simulink model "doa_diego"  (R2013b)
settings: Solver=ode45  StartTime=0.0  StopTime=10.0  RelTol=1e-3
undefined symbols (not defined in the file or in its folder): calan_lib, comp_addsub, pfb_coeff_gen_calc
libraries: xbsIndex_r4 x26847, casper_library_misc x201, casper_library_delays x104, dspsigops x16, xbsReplacements_r4 x4, casper_library_pfbs x4, simulink x2, xps_library x1
(part 2 of 280)

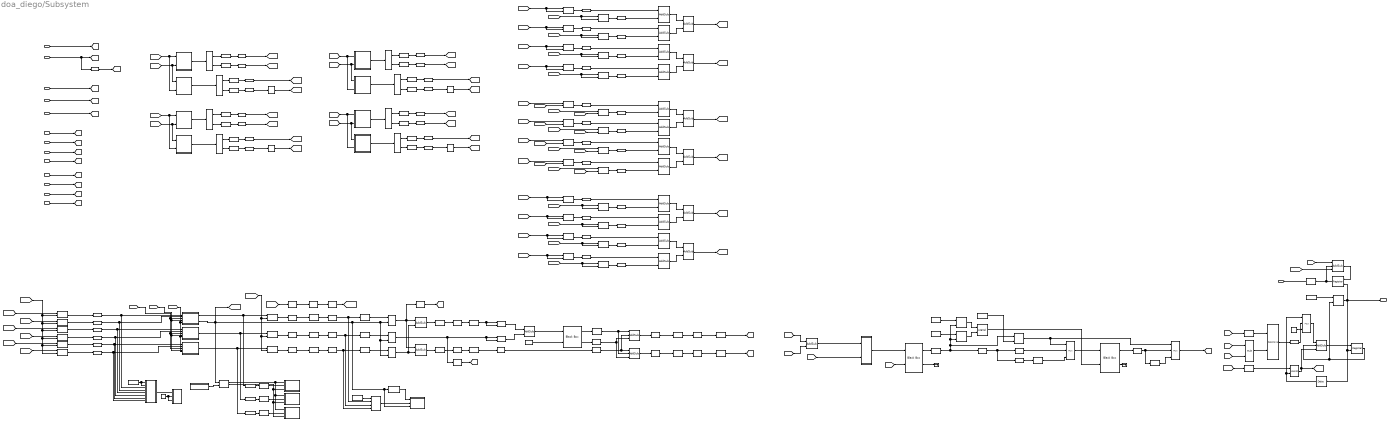
== system doa_diego/Subsystem ==
  gain1 : Inport
  gain2 : Inport  Port=2
  nacc : Inport  Port=3
  snr : Inport  Port=4
  en : Inport  Port=5
  a1_ch0 : Inport  Port=6
  a1_ch1 : Inport  Port=7
  a1_ch2 : Inport  Port=8
  a1_ch3 : Inport  Port=9
  a2_ch0 : Inport  Port=10
  a2_ch1 : Inport  Port=11
  a2_ch2 : Inport  Port=12
  a2_ch3 : Inport  Port=13
  AddSub : xbsIndex_r4/AddSub  mode=Addition  use_carryin=off  use_carryout=off  en=on  latency=1  precision=User Defined  arith_type="Signed  (2's comp)"  n_bits=26  bin_pt=8  quantization="Round  (unbiased: +/- Inf)"  overflow=Saturate  dbl_ovrd=off  use_behavioral_HDL=off  hw_selection=Fabric  pipelined=off  block_type=addsub
  AddSub1 : xbsIndex_r4/AddSub  mode=Addition  use_carryin=off  use_carryout=off  en=on  latency=1  precision=User Defined  arith_type=Unsigned  n_bits=10  bin_pt=0  quantization="Round  (unbiased: +/- Inf)"  overflow=Saturate  dbl_ovrd=off  use_behavioral_HDL=off  hw_selection=Fabric  pipelined=off  block_type=addsub
  AddSub{2..5,8..11,14..15,18..19} (12 alike) : xbsIndex_r4/AddSub  mode=Addition  use_carryin=off  use_carryout=off  en=off  latency=0  precision=Full  arith_type="Signed  (2's comp)"  n_bits=9  bin_pt=7  quantization="Round  (unbiased: +/- Inf)"  overflow=Saturate  dbl_ovrd=off  use_behavioral_HDL=off  hw_selection=Fabric  pipelined=off  block_type=addsub
  AddSub{6..7,12..13,16..17} (6 alike) : xbsIndex_r4/AddSub  mode=Addition  use_carryin=off  use_carryout=off  en=off  latency=0  precision=Full  arith_type="Signed  (2's comp)"  n_bits=10  bin_pt=7  quantization="Round  (unbiased: +/- Inf)"  overflow=Saturate  dbl_ovrd=off  use_behavioral_HDL=off  hw_selection=Fabric  pipelined=off  block_type=addsub
  Black Box : xbsIndex_r4/Black Box  init_code=arcsin_config  sim_method=ISE Simulator  verbose=off  block_type=blackbox2
  Constant : xbsIndex_r4/Constant  const=0  gui_display_data_type=Fixed-point  arith_type=Unsigned  n_bits=8  bin_pt=0  preci_type=Single  exp_width=8  frac_width=24  explicit_period=on  period=1  equ=P=C  opselect=C  inp2=PCIN>>17  opr=+  inp1=P  carry=CIN  dbl_ovrd=off  block_type=constant
  Constant1 : xbsIndex_r4/Constant  const=1  gui_display_data_type=Boolean  arith_type=Boolean  n_bits=16  bin_pt=14  preci_type=Single  exp_width=8  frac_width=24  explicit_period=on  period=1  equ=P=C  opselect=C  inp2=PCIN>>17  opr=+  inp1=P  carry=CIN  dbl_ovrd=off  block_type=constant
  Constant16 : xbsIndex_r4/Constant  const=0  gui_display_data_type=Fixed-point  arith_type=Signed (2's comp)  n_bits=16  bin_pt=12  preci_type=Single  exp_width=8  frac_width=24  explicit_period=on  period=1  equ=P=C  opselect=C  inp2=PCIN>>17  opr=+  inp1=P  carry=CIN  dbl_ovrd=off  block_type=constant
  Constant17 : xbsIndex_r4/Constant  const=16  gui_display_data_type=Fixed-point  arith_type=Unsigned  n_bits=10  bin_pt=0  preci_type=Single  exp_width=8  frac_width=24  explicit_period=on  period=1  equ=P=C  opselect=C  inp2=PCIN>>17  opr=+  inp1=P  carry=CIN  dbl_ovrd=off  block_type=constant
  Constant18 : xbsIndex_r4/Constant  const=0  gui_display_data_type=Fixed-point  arith_type=Unsigned  n_bits=1  bin_pt=0  preci_type=Single  exp_width=8  frac_width=24  explicit_period=on  period=1  equ=P=C  opselect=C  inp2=PCIN>>17  opr=+  inp1=P  carry=CIN  dbl_ovrd=off  block_type=constant
  Constant2 : xbsIndex_r4/Constant  const=0  gui_display_data_type=Fixed-point  arith_type=Signed (2's comp)  n_bits=8  bin_pt=7  preci_type=Single  exp_width=8  frac_width=24  explicit_period=on  period=1  equ=P=C  opselect=C  inp2=PCIN>>17  opr=+  inp1=P  carry=CIN  dbl_ovrd=off  block_type=constant
  Constant6 : xbsIndex_r4/Constant  const=1  gui_display_data_type=Boolean  arith_type=Boolean  n_bits=16  bin_pt=14  preci_type=Single  exp_width=8  frac_width=24  explicit_period=off  period=1  equ=P=C  opselect=C  inp2=PCIN>>17  opr=+  inp1=P  carry=CIN  dbl_ovrd=off  block_type=constant
  Constant7 : xbsIndex_r4/Constant  const=1  gui_display_data_type=Fixed-point  arith_type=Signed (2's comp)  n_bits=16  bin_pt=12  preci_type=Single  exp_width=8  frac_width=24  explicit_period=on  period=1  equ=P=C  opselect=C  inp2=PCIN>>17  opr=+  inp1=P  carry=CIN  dbl_ovrd=off  block_type=constant
  Constant9 : xbsIndex_r4/Constant  const=-1  gui_display_data_type=Fixed-point  arith_type=Signed (2's comp)  n_bits=16  bin_pt=12  preci_type=Single  exp_width=8  frac_width=24  explicit_period=on  period=1  equ=P=C  opselect=C  inp2=PCIN>>17  opr=+  inp1=P  carry=CIN  dbl_ovrd=off  block_type=constant
  Convert{,1..7,15,29..34,41,46..49,54,59..64} (27 alike) : xbsIndex_r4/Convert  gui_display_data_type=Fixed-point  arith_type="Signed  (2's comp)"  n_bits=8  bin_pt=7  float_type=Single  exp_bits=8  fraction_bits=24  quantization="Round  (unbiased: +/- Inf)"  overflow=Saturate  en=off  latency=0  dbl_ovrd=off  pipeline=off  block_type=convert
  Convert10 : xbsIndex_r4/Convert  gui_display_data_type=Fixed-point  arith_type="Signed  (2's comp)"  n_bits=32  bin_pt=7  float_type=Single  exp_bits=8  fraction_bits=24  quantization=Truncate  overflow=Wrap  en=off  latency=0  dbl_ovrd=off  pipeline=off  block_type=convert
      settings: block_version=11.4
  Convert{11..14,20..25,66..75,82..83,93..94} (24 alike) : xbsIndex_r4/Convert  gui_display_data_type=Fixed-point  arith_type="Signed  (2's comp)"  n_bits=15  bin_pt=14  float_type=Single  exp_bits=8  fraction_bits=24  quantization="Round  (unbiased: +/- Inf)"  overflow=Saturate  en=off  latency=0  dbl_ovrd=off  pipeline=off  block_type=convert
  Convert16 : xbsIndex_r4/Convert  gui_display_data_type=Fixed-point  arith_type="Signed  (2's comp)"  n_bits=32  bin_pt=7  float_type=Single  exp_bits=8  fraction_bits=24  quantization=Truncate  overflow=Wrap  en=off  latency=0  dbl_ovrd=off  pipeline=off  block_type=convert
      settings: block_version=11.4
  Convert26 : xbsIndex_r4/Convert  gui_display_data_type=Fixed-point  arith_type="Signed  (2's comp)"  n_bits=15  bin_pt=6  float_type=Single  exp_bits=8  fraction_bits=24  quantization="Round  (unbiased: +/- Inf)"  overflow=Saturate  en=off  latency=0  dbl_ovrd=off  pipeline=off  block_type=convert
  Convert27 : xbsIndex_r4/Convert  gui_display_data_type=Fixed-point  arith_type="Signed  (2's comp)"  n_bits=9  bin_pt=8  float_type=Single  exp_bits=8  fraction_bits=24  quantization="Round  (unbiased: +/- Inf)"  overflow=Saturate  en=off  latency=0  dbl_ovrd=off  pipeline=off  block_type=convert
  Convert28 : xbsIndex_r4/Convert  gui_display_data_type=Fixed-point  arith_type="Signed  (2's comp)"  n_bits=13  bin_pt=12  float_type=Single  exp_bits=8  fraction_bits=24  quantization="Round  (unbiased: +/- Inf)"  overflow=Saturate  en=off  latency=0  dbl_ovrd=off  pipeline=off  block_type=convert
  Convert35 : xbsIndex_r4/Convert  gui_display_data_type=Fixed-point  arith_type="Signed  (2's comp)"  n_bits=28  bin_pt=7  float_type=Single  exp_bits=8  fraction_bits=24  quantization="Round  (unbiased: +/- Inf)"  overflow=Saturate  en=off  latency=0  dbl_ovrd=off  pipeline=off  block_type=convert
  Convert36 : xbsIndex_r4/Convert  gui_display_data_type=Fixed-point  arith_type="Signed  (2's comp)"  n_bits=28  bin_pt=7  float_type=Single  exp_bits=8  fraction_bits=24  quantization="Round  (unbiased: +/- Inf)"  overflow=Saturate  en=off  latency=0  dbl_ovrd=off  pipeline=off  block_type=convert
  Convert37 : xbsIndex_r4/Convert  gui_display_data_type=Fixed-point  arith_type="Signed  (2's comp)"  n_bits=28  bin_pt=7  float_type=Single  exp_bits=8  fraction_bits=24  quantization="Round  (unbiased: +/- Inf)"  overflow=Saturate  en=off  latency=0  dbl_ovrd=off  pipeline=off  block_type=convert
  Convert38 : xbsIndex_r4/Convert  gui_display_data_type=Fixed-point  arith_type="Signed  (2's comp)"  n_bits=15  bin_pt=6  float_type=Single  exp_bits=8  fraction_bits=24  quantization="Round  (unbiased: +/- Inf)"  overflow=Saturate  en=off  latency=0  dbl_ovrd=off  pipeline=off  block_type=convert
  Convert39 : xbsIndex_r4/Convert  gui_display_data_type=Fixed-point  arith_type="Signed  (2's comp)"  n_bits=9  bin_pt=8  float_type=Single  exp_bits=8  fraction_bits=24  quantization="Round  (unbiased: +/- Inf)"  overflow=Saturate  en=off  latency=0  dbl_ovrd=off  pipeline=off  block_type=convert
  Convert40 : xbsIndex_r4/Convert  gui_display_data_type=Fixed-point  arith_type=Unsigned  n_bits=1  bin_pt=0  float_type=Single  exp_bits=8  fraction_bits=24  quantization="Round  (unbiased: +/- Inf)"  overflow=Saturate  en=off  latency=0  dbl_ovrd=off  pipeline=off  block_type=convert
  Convert8 : xbsIndex_r4/Convert  gui_display_data_type=Boolean  arith_type=Boolean  n_bits=8  bin_pt=0  float_type=Single  exp_bits=8  fraction_bits=24  quantization="Round  (unbiased: +/- Inf)"  overflow=Saturate  en=off  latency=0  dbl_ovrd=off  pipeline=off  block_type=convert
  Convert9 : xbsIndex_r4/Convert  gui_display_data_type=Fixed-point  arith_type="Signed  (2's comp)"  n_bits=32  bin_pt=7  float_type=Single  exp_bits=8  fraction_bits=24  quantization=Truncate  overflow=Wrap  en=off  latency=0  dbl_ovrd=off  pipeline=off  block_type=convert
      settings: block_version=11.4
  Counter : xbsIndex_r4/Counter  cnt_type=Free Running  cnt_to=Inf  operation=Up  start_count=0  cnt_by_val=1  arith_type=Unsigned  n_bits=10  bin_pt=0  load_pin=off  rst=off  en=on  explicit_period=on  period=1  dbl_ovrd=off  use_behavioral_HDL=off  implementation=Fabric  block_type=counter
  Delay : xbsIndex_r4/Delay  rst=off  infoeditControl=Selection of Reset will increase slice count due to use of real FFs and instead of SRLs  en=off  latency=3  dbl_ovrd=off  reg_retiming=off  block_type=delay
  Delay1 : xbsIndex_r4/Delay  rst=off  infoeditControl=Selection of Reset will increase slice count due to use of real FFs and instead of SRLs  en=off  latency=1  dbl_ovrd=off  reg_retiming=off  block_type=delay
  Delay11 : xbsIndex_r4/Delay  rst=off  infoeditControl=Selection of Reset will increase slice count due to use of real FFs and instead of SRLs  en=off  latency=3  dbl_ovrd=off  reg_retiming=off  block_type=delay
  Delay{4,14,16,20..21} (5 alike) : xbsIndex_r4/Delay  rst=off  infoeditControl=Selection of Reset will increase slice count due to use of real FFs and instead of SRLs  en=off  latency=4  dbl_ovrd=off  reg_retiming=off  block_type=delay
  Delay2 : xbsIndex_r4/Delay  rst=off  infoeditControl=Selection of Reset will increase slice count due to use of real FFs and instead of SRLs  en=off  latency=3  dbl_ovrd=off  reg_retiming=off  block_type=delay
  Delay22 : xbsIndex_r4/Delay  rst=off  infoeditControl=Selection of Reset will increase slice count due to use of real FFs and instead of SRLs  en=off  latency=1  dbl_ovrd=off  reg_retiming=off  block_type=delay
  Logical : xbsIndex_r4/Logical  logical_function=OR  inputs=2  en=off  latency=0  precision=Full  arith_type=Unsigned  n_bits=16  bin_pt=0  align_bp=on  dbl_ovrd=off  block_type=logical
  Logical1 : xbsIndex_r4/Logical  logical_function=AND  inputs=2  en=off  latency=0  precision=Full  arith_type=Unsigned  n_bits=16  bin_pt=0  align_bp=on  dbl_ovrd=off  block_type=logical
  Mult{,0..11,14..34} (34 alike) : xbsIndex_r4/Mult  precision=Full  arith_type="Signed  (2's comp)"  n_bits=16  bin_pt=14  quantization=Truncate  overflow=Wrap  en=off  latency=3  dbl_ovrd=off  use_behavioral_HDL=off  opt=Speed  use_embedded=on  optimum_pipeline=off  pipeline=on  use_rpm=on  placement_style=Triangular  block_type=mult
  Mult12 : xbsIndex_r4/Mult  precision=User Defined  arith_type=Unsigned  n_bits=16  bin_pt=0  quantization="Round  (unbiased: +/- Inf)"  overflow=Saturate  en=off  latency=3  dbl_ovrd=off  use_behavioral_HDL=off  opt=Speed  use_embedded=on  optimum_pipeline=off  pipeline=on  use_rpm=on  placement_style=Triangular  block_type=mult
  Mult13 : xbsIndex_r4/Mult  precision=User Defined  arith_type=Unsigned  n_bits=16  bin_pt=0  quantization="Round  (unbiased: +/- Inf)"  overflow=Saturate  en=off  latency=3  dbl_ovrd=off  use_behavioral_HDL=off  opt=Speed  use_embedded=on  optimum_pipeline=off  pipeline=on  use_rpm=on  placement_style=Triangular  block_type=mult
  Mux : xbsIndex_r4/Mux  inputs=2  en=off  latency=0  precision=Full  arith_type=Unsigned  n_bits=16  bin_pt=14  quantization=Truncate  overflow=Wrap  dbl_ovrd=off  block_type=mux
  Mux1 : xbsIndex_r4/Mux  inputs=2  en=off  latency=0  precision=Full  arith_type=Unsigned  n_bits=16  bin_pt=14  quantization=Truncate  overflow=Wrap  dbl_ovrd=off  block_type=mux
  Mux2 : xbsIndex_r4/Mux  inputs=2  en=off  latency=0  precision=Full  arith_type=Unsigned  n_bits=16  bin_pt=14  quantization=Truncate  overflow=Wrap  dbl_ovrd=off  block_type=mux
  Mx11 : xbsIndex_r4/Register  init=0  rst=off  en=on  dbl_ovrd=off  block_type=register
  Mx12 : xbsIndex_r4/Register  init=0  rst=off  en=on  dbl_ovrd=off  block_type=register
  Mx22 : xbsIndex_r4/Register  init=0  rst=off  en=on  dbl_ovrd=off  block_type=register
  Negate{,4,6,8} (4 alike) : xbsIndex_r4/Negate  precision=User Defined  arith_type="Signed  (2's comp)"  n_bits=8  bin_pt=7  quantization="Round  (unbiased: +/- Inf)"  overflow=Saturate  en=off  latency=0  dbl_ovrd=off  block_type=negate
  Negate2 : xbsIndex_r4/Negate  precision=User Defined  arith_type="Signed  (2's comp)"  n_bits=13  bin_pt=12  quantization="Round  (unbiased: +/- Inf)"  overflow=Saturate  en=off  latency=0  dbl_ovrd=off  block_type=negate
  Negate3 : xbsIndex_r4/Negate  precision=User Defined  arith_type="Signed  (2's comp)"  n_bits=16  bin_pt=8  quantization="Round  (unbiased: +/- Inf)"  overflow=Saturate  en=off  latency=0  dbl_ovrd=off  block_type=negate
  Register : xbsIndex_r4/Register  init=0  rst=on  en=off  dbl_ovrd=off  block_type=register
  Register1 : xbsIndex_r4/Register  init=0  rst=on  en=off  dbl_ovrd=off  block_type=register
  Reinterpret : xbsIndex_r4/Reinterpret  force_arith_type=off  arith_type=Unsigned  force_bin_pt=on  bin_pt=6  block_type=reinterpret
  Reinterpret1 : xbsIndex_r4/Reinterpret  force_arith_type=off  arith_type=Unsigned  force_bin_pt=off  bin_pt=0  block_type=reinterpret
  Reinterpret10 : xbsIndex_r4/Reinterpret  force_arith_type=on  arith_type="Signed  (2's comp)"  force_bin_pt=on  bin_pt=0  block_type=reinterpret
  Reinterpret{7..9,11..14,22..23,37..41,43..45} (17 alike) : xbsIndex_r4/Reinterpret  force_arith_type=on  arith_type="Signed  (2's comp)"  force_bin_pt=on  bin_pt=8  block_type=reinterpret
  Reinterpret15 : xbsIndex_r4/Reinterpret  force_arith_type=off  arith_type=Unsigned  force_bin_pt=on  bin_pt=14  block_type=reinterpret
  Reinterpret{6,16,21,42} (4 alike) : xbsIndex_r4/Reinterpret  force_arith_type=off  arith_type=Unsigned  force_bin_pt=on  bin_pt=0  block_type=reinterpret
  Reinterpret17 : xbsIndex_r4/Reinterpret  force_arith_type=on  arith_type="Signed  (2's comp)"  force_bin_pt=on  bin_pt=12  block_type=reinterpret
  Reinterpret18 : xbsIndex_r4/Reinterpret  force_arith_type=on  arith_type=Unsigned  force_bin_pt=on  bin_pt=0  block_type=reinterpret
  Reinterpret19 : xbsIndex_r4/Reinterpret  force_arith_type=on  arith_type=Unsigned  force_bin_pt=on  bin_pt=0  block_type=reinterpret
  Reinterpret2 : xbsIndex_r4/Reinterpret  force_arith_type=off  arith_type=Unsigned  force_bin_pt=off  bin_pt=0  block_type=reinterpret
  Reinterpret20 : xbsIndex_r4/Reinterpret  force_arith_type=off  arith_type=Unsigned  force_bin_pt=on  bin_pt=14  block_type=reinterpret
  Reinterpret3 : xbsIndex_r4/Reinterpret  force_arith_type=off  arith_type=Unsigned  force_bin_pt=off  bin_pt=0  block_type=reinterpret
  Reinterpret30 : xbsIndex_r4/Reinterpret  force_arith_type=off  arith_type=Unsigned  force_bin_pt=on  bin_pt=8  block_type=reinterpret
  Reinterpret5 : xbsIndex_r4/Reinterpret  force_arith_type=off  arith_type=Unsigned  force_bin_pt=on  bin_pt=8  block_type=reinterpret
  Reinterpret53 : xbsIndex_r4/Reinterpret  force_arith_type=on  arith_type="Signed  (2's comp)"  force_bin_pt=on  bin_pt=27  block_type=reinterpret
  Reinterpret64 : xbsIndex_r4/Reinterpret  force_arith_type=on  arith_type="Signed  (2's comp)"  force_bin_pt=on  bin_pt=0  block_type=reinterpret
  Reinterpret75 : xbsIndex_r4/Reinterpret  force_arith_type=on  arith_type="Signed  (2's comp)"  force_bin_pt=on  bin_pt=27  block_type=reinterpret
  Reinterpret76 : xbsIndex_r4/Reinterpret  force_arith_type=on  arith_type="Signed  (2's comp)"  force_bin_pt=on  bin_pt=0  block_type=reinterpret
  Reinterpret77 : xbsIndex_r4/Reinterpret  force_arith_type=on  arith_type="Signed  (2's comp)"  force_bin_pt=on  bin_pt=27  block_type=reinterpret
  Relational : xbsIndex_r4/Relational  mode=a>=b  en=off  latency=0  dbl_ovrd=off  block_type=relational
  Relational1 : xbsIndex_r4/Relational  mode=a<=b  en=off  latency=0  dbl_ovrd=off  block_type=relational
  Relational2 : xbsIndex_r4/Relational  mode=a<=b  en=off  latency=0  dbl_ovrd=off  block_type=relational
  Relational3 : xbsIndex_r4/Relational  mode=a>b  en=off  latency=0  dbl_ovrd=off  block_type=relational
  Relational4 : xbsIndex_r4/Relational  mode=a=b  en=off  latency=0  dbl_ovrd=off  block_type=relational
  Shift : xbsIndex_r4/Shift  shift_dir=Right  shift_bits=4  en=off  latency=0  precision=Full  arith_type="Signed  (2's comp)"  n_bits=16  bin_pt=14  quantization=Truncate  overflow=Wrap  dbl_ovrd=off  block_type=shift
  Subsystem16 : Subsystem
  Subsystem17 : Subsystem
  Subsystem5 : Subsystem
  Terminator : Terminator
  Terminator2 : Terminator
  a_c1 : xbsIndex_r4/AddSub  mode=Subtraction  use_carryin=off  use_carryout=off  en=off  latency=0  precision=Full  arith_type="Signed  (2's comp)"  n_bits=64  bin_pt=26  quantization="Round  (unbiased: +/- Inf)"  overflow=Wrap  dbl_ovrd=off  use_behavioral_HDL=off  hw_selection=Fabric  pipelined=off  block_type=addsub
  arctan : xbsIndex_r4/Black Box  init_code=arctan2_config  sim_method=ISE Simulator  verbose=off  block_type=blackbox2
  bus_create : Subsystem  AncestorBlock=casper_library_flow_control/bus_create  mask(bus_create){inputNum=8}
      mask prompts: inputNum "Number of inputs:"
      mask init:
      | bus_create_init(gcb,...
      |     'inputNum', inputNum);
  bus_create1 : Subsystem  AncestorBlock=casper_library_flow_control/bus_create  mask(bus_create){inputNum=4}
      mask prompts: inputNum "Number of inputs:"
      mask init:
      | bus_create_init(gcb,...
      |     'inputNum', inputNum);
  bus_create2 : Subsystem  AncestorBlock=casper_library_flow_control/bus_create  mask(bus_create){inputNum=2}
      mask prompts: inputNum "Number of inputs:"
      mask init:
      | bus_create_init(gcb,...
      |     'inputNum', inputNum);
  c_to_ri{,1,4..5,8..9,12..13} (8 alike) : casper_library_misc/c_to_ri  n_bits=9  bin_pt=7
      settings: AttributesFormatString=9_7 r/i
  comp_addsub : Subsystem  AncestorBlock=calan_lib/comp_addsub  mask(comp_addsub){addsub=Addition, n_bits_in=8, bin_pt_in=7, add_latency=2, n_bits_out=9, bin_pt_out=7, quantization=Round  (unbiased: +/- Inf), overflow=Saturate}
      mask prompts: addsub "Addition/Subtraction"; n_bits_in "Bit Width"; bin_pt_in "Binary Point"; add_latency "Adder Latency"; n_bits_out "Bit Width"; bin_pt_out "BInary Point"
      mask help: Add or substract two complex numbers of the same length.
      mask init:
      | comp_addsub_init(gcb, ...
      |     'addsub', addsub, ...
      |     'n_bits_in', n_bits_in, ...
      |     'bin_pt_in', bin_pt_in, ...
      |     'n_bits_out', n_bits_out, ...
      |     'bin_pt_out', bin_pt_out, ...
      |     'quantization', quantization, ...
      |     'overflow', overflow, ...
      |     'add_latency', add_latency);
      settings: AttributesFormatString=|
      | 8_7 * 8_7 ==> 9_7
      | Round  (unbiased: +/- Inf), Saturate
      mask TypeOptions addsub: Addition | Subtraction
      mask TypeOptions quantization: Truncate | Round  (unbiased: +/- Inf)
      mask TypeOptions overflow: Wrap | Saturate | Flag as error
      mask Display:
      | port_label('input', 1, 'a');
      | port_label('input', 2, 'b');
      | if strcmp(addsub, 'Addition')
      |     port_label('output', 1, 'a+b');
      | elseif strcmp(addsub, 'Subtraction')
      |     port_label('output', 1, 'a-b');
      | end
      | disp(['Z^-', num2str(add_latency)]);
  comp_addsub1 : Subsystem  AncestorBlock=calan_lib/comp_addsub  mask(comp_addsub){addsub=Subtraction, n_bits_in=8, bin_pt_in=7, add_latency=2, n_bits_out=9, bin_pt_out=7, quantization=Round  (unbiased: +/- Inf), overflow=Saturate}
      mask prompts: addsub "Addition/Subtraction"; n_bits_in "Bit Width"; bin_pt_in "Binary Point"; add_latency "Adder Latency"; n_bits_out "Bit Width"; bin_pt_out "BInary Point"
      mask help: Add or substract two complex numbers of the same length.
      mask init: same as doa_diego/Subsystem/comp_addsub
      settings: AttributesFormatString=|
      | 8_7 * 8_7 ==> 9_7
      | Round  (unbiased: +/- Inf), Saturate
      mask TypeOptions addsub: Addition | Subtraction
      mask TypeOptions quantization: Truncate | Round  (unbiased: +/- Inf)
      mask TypeOptions overflow: Wrap | Saturate | Flag as error
      mask Display:
      | port_label('input', 1, 'a');
      | port_label('input', 2, 'b');
      | if strcmp(addsub, 'Addition')
      |     port_label('output', 1, 'a+b');
      | elseif strcmp(addsub, 'Subtraction')
      |     port_label('output', 1, 'a-b');
      | end
      | disp(['Z^-', num2str(add_latency)]);
  comp_addsub12 : Subsystem  AncestorBlock=calan_lib/comp_addsub  mask(comp_addsub){addsub=Addition, n_bits_in=8, bin_pt_in=7, add_latency=2, n_bits_out=9, bin_pt_out=7, quantization=Round  (unbiased: +/- Inf), overflow=Saturate}
      mask prompts: addsub "Addition/Subtraction"; n_bits_in "Bit Width"; bin_pt_in "Binary Point"; add_latency "Adder Latency"; n_bits_out "Bit Width"; bin_pt_out "BInary Point"
      mask help: Add or substract two complex numbers of the same length.
      mask init: same as doa_diego/Subsystem/comp_addsub
      settings: AttributesFormatString=|
      | 8_7 * 8_7 ==> 9_7
      | Round  (unbiased: +/- Inf), Saturate
      mask TypeOptions addsub: Addition | Subtraction
      mask TypeOptions quantization: Truncate | Round  (unbiased: +/- Inf)
      mask TypeOptions overflow: Wrap | Saturate | Flag as error
      mask Display:
      | port_label('input', 1, 'a');
      | port_label('input', 2, 'b');
      | if strcmp(addsub, 'Addition')
      |     port_label('output', 1, 'a+b');
      | elseif strcmp(addsub, 'Subtraction')
      |     port_label('output', 1, 'a-b');
      | end
      | disp(['Z^-', num2str(add_latency)]);
  comp_addsub13 : Subsystem  AncestorBlock=calan_lib/comp_addsub  mask(comp_addsub){addsub=Subtraction, n_bits_in=8, bin_pt_in=7, add_latency=2, n_bits_out=9, bin_pt_out=7, quantization=Round  (unbiased: +/- Inf), overflow=Saturate}
      mask prompts: addsub "Addition/Subtraction"; n_bits_in "Bit Width"; bin_pt_in "Binary Point"; add_latency "Adder Latency"; n_bits_out "Bit Width"; bin_pt_out "BInary Point"
      mask help: Add or substract two complex numbers of the same length.
      mask init: same as doa_diego/Subsystem/comp_addsub
      settings: AttributesFormatString=|
      | 8_7 * 8_7 ==> 9_7
      | Round  (unbiased: +/- Inf), Saturate
      mask TypeOptions addsub: Addition | Subtraction
      mask TypeOptions quantization: Truncate | Round  (unbiased: +/- Inf)
      mask TypeOptions overflow: Wrap | Saturate | Flag as error
      mask Display:
      | port_label('input', 1, 'a');
      | port_label('input', 2, 'b');
      | if strcmp(addsub, 'Addition')
      |     port_label('output', 1, 'a+b');
      | elseif strcmp(addsub, 'Subtraction')
      |     port_label('output', 1, 'a-b');
      | end
      | disp(['Z^-', num2str(add_latency)]);
  comp_addsub4 : Subsystem  AncestorBlock=calan_lib/comp_addsub  mask(comp_addsub){addsub=Addition, n_bits_in=8, bin_pt_in=7, add_latency=2, n_bits_out=9, bin_pt_out=7, quantization=Round  (unbiased: +/- Inf), overflow=Saturate}
      mask prompts: addsub "Addition/Subtraction"; n_bits_in "Bit Width"; bin_pt_in "Binary Point"; add_latency "Adder Latency"; n_bits_out "Bit Width"; bin_pt_out "BInary Point"
      mask help: Add or substract two complex numbers of the same length.
      mask init: same as doa_diego/Subsystem/comp_addsub
      settings: AttributesFormatString=|
      | 8_7 * 8_7 ==> 9_7
      | Round  (unbiased: +/- Inf), Saturate
      mask TypeOptions addsub: Addition | Subtraction
      mask TypeOptions quantization: Truncate | Round  (unbiased: +/- Inf)
      mask TypeOptions overflow: Wrap | Saturate | Flag as error
      mask Display:
      | port_label('input', 1, 'a');
      | port_label('input', 2, 'b');
      | if strcmp(addsub, 'Addition')
      |     port_label('output', 1, 'a+b');
      | elseif strcmp(addsub, 'Subtraction')
      |     port_label('output', 1, 'a-b');
      | end
      | disp(['Z^-', num2str(add_latency)]);
  comp_addsub5 : Subsystem  AncestorBlock=calan_lib/comp_addsub  mask(comp_addsub){addsub=Subtraction, n_bits_in=8, bin_pt_in=7, add_latency=2, n_bits_out=9, bin_pt_out=7, quantization=Round  (unbiased: +/- Inf), overflow=Saturate}
      mask prompts: addsub "Addition/Subtraction"; n_bits_in "Bit Width"; bin_pt_in "Binary Point"; add_latency "Adder Latency"; n_bits_out "Bit Width"; bin_pt_out "BInary Point"
      mask help: Add or substract two complex numbers of the same length.
      mask init: same as doa_diego/Subsystem/comp_addsub
      settings: AttributesFormatString=|
      | 8_7 * 8_7 ==> 9_7
      | Round  (unbiased: +/- Inf), Saturate
      mask TypeOptions addsub: Addition | Subtraction
      mask TypeOptions quantization: Truncate | Round  (unbiased: +/- Inf)
      mask TypeOptions overflow: Wrap | Saturate | Flag as error
      mask Display:
      | port_label('input', 1, 'a');
      | port_label('input', 2, 'b');
      | if strcmp(addsub, 'Addition')
      |     port_label('output', 1, 'a+b');
      | elseif strcmp(addsub, 'Subtraction')
      |     port_label('output', 1, 'a-b');
      | end
      | disp(['Z^-', num2str(add_latency)]);
  comp_addsub8 : Subsystem  AncestorBlock=calan_lib/comp_addsub  mask(comp_addsub){addsub=Addition, n_bits_in=8, bin_pt_in=7, add_latency=2, n_bits_out=9, bin_pt_out=7, quantization=Round  (unbiased: +/- Inf), overflow=Saturate}
      mask prompts: addsub "Addition/Subtraction"; n_bits_in "Bit Width"; bin_pt_in "Binary Point"; add_latency "Adder Latency"; n_bits_out "Bit Width"; bin_pt_out "BInary Point"
      mask help: Add or substract two complex numbers of the same length.
      mask init: same as doa_diego/Subsystem/comp_addsub
      settings: AttributesFormatString=|
      | 8_7 * 8_7 ==> 9_7
      | Round  (unbiased: +/- Inf), Saturate
      mask TypeOptions addsub: Addition | Subtraction
      mask TypeOptions quantization: Truncate | Round  (unbiased: +/- Inf)
      mask TypeOptions overflow: Wrap | Saturate | Flag as error
      mask Display:
      | port_label('input', 1, 'a');
      | port_label('input', 2, 'b');
      | if strcmp(addsub, 'Addition')
      |     port_label('output', 1, 'a+b');
      | elseif strcmp(addsub, 'Subtraction')
      |     port_label('output', 1, 'a-b');
      | end
      | disp(['Z^-', num2str(add_latency)]);
  comp_addsub9 : Subsystem  AncestorBlock=calan_lib/comp_addsub  mask(comp_addsub){addsub=Subtraction, n_bits_in=8, bin_pt_in=7, add_latency=2, n_bits_out=9, bin_pt_out=7, quantization=Round  (unbiased: +/- Inf), overflow=Saturate}
      mask prompts: addsub "Addition/Subtraction"; n_bits_in "Bit Width"; bin_pt_in "Binary Point"; add_latency "Adder Latency"; n_bits_out "Bit Width"; bin_pt_out "BInary Point"
      mask help: Add or substract two complex numbers of the same length.
      mask init: same as doa_diego/Subsystem/comp_addsub
      settings: AttributesFormatString=|
      | 8_7 * 8_7 ==> 9_7
      | Round  (unbiased: +/- Inf), Saturate
      mask TypeOptions addsub: Addition | Subtraction
      mask TypeOptions quantization: Truncate | Round  (unbiased: +/- Inf)
      mask TypeOptions overflow: Wrap | Saturate | Flag as error
      mask Display:
      | port_label('input', 1, 'a');
      | port_label('input', 2, 'b');
      | if strcmp(addsub, 'Addition')
      |     port_label('output', 1, 'a+b');
      | elseif strcmp(addsub, 'Subtraction')
      |     port_label('output', 1, 'a-b');
      | end
      | disp(['Z^-', num2str(add_latency)]);
  corr_mat_red : Subsystem  Tag=xps:bram  AncestorBlock=xps_library/Shared_BRAM  mask{arith_type=Unsigned, addr_width=10, data_width=32, reg_prim_output=off, reg_core_output=off, optimization=Minimum_Area, data_bin_pt=0, init_vals=[0:2^10-1], sample_rate=1}
      mask prompts: arith_type "Output Data Type"; addr_width "Address width"; data_width "Data Width"; reg_prim_output "Register Primitive Output"; reg_core_output "Register Core Output"; data_bin_pt "Data Binary Point"; init_vals "Initial values (simulation only)"; sample_rate "Sample rate"
      mask init:
      | shared_bram_mask;
      mask TypeOptions arith_type: Unsigned | Signed  (2's comp)
      mask TypeOptions data_width: 8 | 16 | 32 | 64 | 128
      mask TypeOptions optimization: Minimum_Area | Low_Power
  edge_detect1 : casper_library_misc/edge_detect  edge=Rising  polarity=Active High  x_in=[0 0.08 0.08 0.16 0.16 0.24 0.24 0.32 0.32 0.4]  y_in="[-1 -1  1  1  1  1  1  1  1  1]"  x_out=[0.6 0.68 0.68 0.76 0.76 0.84 0.84 0.92 0.92 1]  y_out="[-1 -1  1  1 -1 -1 -1 -1 -1 -1]"
  freeze_cntr : casper_library_misc/freeze_cntr  CounterBits=10
  r1 : Subsystem  Tag=xps:bram  AncestorBlock=xps_library/Shared_BRAM  mask{arith_type=Unsigned, addr_width=10, data_width=32, reg_prim_output=off, reg_core_output=off, optimization=Minimum_Area, data_bin_pt=0, init_vals=[0:2^10-1], sample_rate=1}
      mask prompts: arith_type "Output Data Type"; addr_width "Address width"; data_width "Data Width"; reg_prim_output "Register Primitive Output"; reg_core_output "Register Core Output"; data_bin_pt "Data Binary Point"; init_vals "Initial values (simulation only)"; sample_rate "Sample rate"
      mask init:
      | shared_bram_mask;
      mask TypeOptions arith_type: Unsigned | Signed  (2's comp)
      mask TypeOptions data_width: 8 | 16 | 32 | 64 | 128
      mask TypeOptions optimization: Minimum_Area | Low_Power
  r11 : Subsystem  Tag=xps:bram  AncestorBlock=xps_library/Shared_BRAM  mask{arith_type=Unsigned, addr_width=10, data_width=32, reg_prim_output=off, reg_core_output=off, optimization=Minimum_Area, data_bin_pt=0, init_vals=[0:2^10-1], sample_rate=1}
      mask prompts: arith_type "Output Data Type"; addr_width "Address width"; data_width "Data Width"; reg_prim_output "Register Primitive Output"; reg_core_output "Register Core Output"; data_bin_pt "Data Binary Point"; init_vals "Initial values (simulation only)"; sample_rate "Sample rate"
      mask init:
      | shared_bram_mask;
      mask TypeOptions arith_type: Unsigned | Signed  (2's comp)
      mask TypeOptions data_width: 8 | 16 | 32 | 64 | 128
      mask TypeOptions optimization: Minimum_Area | Low_Power
  r12 : Subsystem  Tag=xps:bram  AncestorBlock=xps_library/Shared_BRAM  mask{arith_type=Unsigned, addr_width=10, data_width=32, reg_prim_output=off, reg_core_output=off, optimization=Minimum_Area, data_bin_pt=0, init_vals=[0:2^10-1], sample_rate=1}
      mask prompts: arith_type "Output Data Type"; addr_width "Address width"; data_width "Data Width"; reg_prim_output "Register Primitive Output"; reg_core_output "Register Core Output"; data_bin_pt "Data Binary Point"; init_vals "Initial values (simulation only)"; sample_rate "Sample rate"
      mask init:
      | shared_bram_mask;
      mask TypeOptions arith_type: Unsigned | Signed  (2's comp)
      mask TypeOptions data_width: 8 | 16 | 32 | 64 | 128
      mask TypeOptions optimization: Minimum_Area | Low_Power
  snapshot : Subsystem  Tag=casper:snapshot  AncestorBlock=casper_library_scopes/snapshot  mask(snapshot){storage=bram, dram_dimm=1, dram_clock=200, nsamples=10, data_width=64, offset=on, circap=off, value=off, use_dsp48=off}
      mask prompts: storage "Storage medium"; dram_dimm "DRAM dimm"; dram_clock "DRAM clock rate"; nsamples "Number of Samples (2^?)"; data_width "Data width"; offset "Start delay support"; circap "Circular capture support"; value "Extra value capture support"; use_dsp48 "Use DSP48s to implement counters"
      mask help:
      | Standard snap block, but with the following optional extras;
      |  1) Optionally delay the fabric trigger by a user-specified number of valids.
      |  2) Capture continuously after a trigger until a stop pulse is received. Data is captured into BRAM continuously, with old data being overwritten until a stop command is received. Then stop capturing when memory is filled. This enables you to get some data before and some data after the stop pulse. Use the 'tr_en_cnt' register to determine where you are in your vector. 'Addr' register's MSb now indicates 'busy capturing'.
      |  3) Optionally capture a value on an input port to a register at the same time as the first data sample.
      |  4) Implement counters using DSP48Es to save resources.
      mask init: same as doa_diego/adcsnap0
      mask TypeOptions storage: bram | dram
      mask Callback storage: snapshot_callback();
      mask TypeOptions dram_dimm: 1 | 2 | 3 | 4
      mask TypeOptions dram_clock: 200 | 250 | 266 | 300 | 333
      mask TypeOptions data_width: 8 | 16 | 32 | 64 | 128
  sqrt : xbsIndex_r4/Black Box  init_code=sqrt_config  sim_method=ISE Simulator  verbose=off  block_type=blackbox2
  tracex1 : xbsIndex_r4/AddSub  mode=Addition  use_carryin=off  use_carryout=off  en=off  latency=0  precision=Full  arith_type="Signed  (2's comp)"  n_bits=64  bin_pt=26  quantization="Round  (unbiased: +/- Inf)"  overflow=Wrap  dbl_ovrd=off  use_behavioral_HDL=off  hw_selection=Fabric  pipelined=off  block_type=addsub
  tracex2 : xbsIndex_r4/AddSub  mode=Addition  use_carryin=off  use_carryout=off  en=off  latency=0  precision=Full  arith_type=Unsigned  n_bits=8  bin_pt=0  quantization="Round  (unbiased: +/- Inf)"  overflow=Saturate  dbl_ovrd=off  use_behavioral_HDL=off  hw_selection=Fabric  pipelined=off  block_type=addsub
  tracex3 : xbsIndex_r4/AddSub  mode=Addition  use_carryin=off  use_carryout=off  en=on  latency=1  precision=Full  arith_type="Signed  (2's comp)"  n_bits=64  bin_pt=26  quantization="Round  (unbiased: +/- Inf)"  overflow=Wrap  dbl_ovrd=off  use_behavioral_HDL=off  hw_selection=Fabric  pipelined=off  block_type=addsub
  tracex4 : xbsIndex_r4/AddSub  mode=Subtraction  use_carryin=off  use_carryout=off  en=on  latency=1  precision=Full  arith_type="Signed  (2's comp)"  n_bits=64  bin_pt=26  quantization="Round  (unbiased: +/- Inf)"  overflow=Wrap  dbl_ovrd=off  use_behavioral_HDL=off  hw_selection=Fabric  pipelined=off  block_type=addsub
  tracex5 : xbsIndex_r4/AddSub  mode=Subtraction  use_carryin=off  use_carryout=off  en=off  latency=1  precision=Full  arith_type="Signed  (2's comp)"  n_bits=64  bin_pt=26  quantization="Round  (unbiased: +/- Inf)"  overflow=Wrap  dbl_ovrd=off  use_behavioral_HDL=off  hw_selection=Fabric  pipelined=off  block_type=addsub
  trig : Subsystem  Tag=xps:sw_reg  AncestorBlock=xps_library/software_register  mask(swreg){io_dir=From Processor, io_delay=0, sample_period=1, names=reg, bitwidths=32, bin_pts=0, arith_types=2, sim_port=on, show_format=on}
      mask prompts: io_dir "I/O direction"; io_delay "I/O delay"; sample_period "Sample period"; names "Bitfield names [msb...lsb]"; bitwidths "Bitfield widths"; bin_pts "Bitfield binary pts"; arith_types "Bitfield types, ufix=0, fix=1, bool=2"; sim_port "Provide sim input/output?"; show_format "Print format string?"
      mask help: A 32-bit software-accessible register. Can be divided into bitfields of varying widths and types using the fields in the block mask.
      mask init:
      | try
      |     swreg_init(gcb)
      | catch ex
      |     disp(sprintf('%s: %s', gcb, ex.message))
      |     errordlg(ex.message)
      | end
      settings: AttributesFormatString=1input:b = 32 bits
      mask TypeOptions io_dir: To Processor | From Processor
      mask Callback io_dir:
      | mask_enables = get_param(gcb, 'MaskEnables');
      | mask_names = get_param(gcb, 'MaskNames');
      | if strcmp(get_param(gcb, 'io_dir'), 'To Processor'),
      |     mask_enables{ismember(mask_names, 'sample_period')} = 'off';
      | else
      |     mask_enables{ismember(mask_names, 'sample_period')} = 'on';
      | end
      | set_param(gcb, 'MaskEnables', mask_enables);
  doa_valid : Outport
  doa_acc : Outport  Port=2
  wires:
  [cx_ch0] -> comp_addsub/2(b), comp_addsub1/1(a)
  Reinterpret11/1 -> Convert5/1
  Convert5/1 -> [x1re_ch0]
  Convert6/1 -> [x1im_ch0]
  Reinterpret12/1 -> Convert6/1
  Reinterpret13/1 -> Convert7/1
  Convert15/1 -> Negate/1
  Reinterpret9/1 -> Convert15/1
  Negate/1 -> [x2re_ch0]
  [ax] -> tracex5/1
  [lamb1x] -> tracex5/2
  Reinterpret15/1 -> Convert27/1
  Convert26/1 -> Reinterpret15/1
  Convert27/1 -> Reinterpret16/1
  Reinterpret16/1 -> [lamb1x]
  bus_create2/1(bus_out) -> arctan/1
  [en] -> arctan/2
  tracex5/1 -> bus_create2/1(in1)
  [bx] -> bus_create2/2(in2)
  arctan/1 -> Reinterpret17/1
  Constant7/1 -> Relational/1
  Constant9/1 -> Relational1/1
  Relational1/1 -> Logical1/1
  Relational/1 -> Logical1/2
  Convert28/1 -> Reinterpret18/1, Negate2/1
  Logical1/1 -> Black Box/2
  Black Box/1 -> Reinterpret14/1
  Reinterpret17/1 -> Convert28/1, Relational2/1, Relational1/2, Relational/2
  Delay4/1 -> [ax]
  c_to_ri13/1 -> Reinterpret43/1
  c_to_ri13/2 -> Reinterpret44/1
  Convert60/1 -> [x1im_ch3]
  Reinterpret41/1 -> Convert60/1
  Reinterpret43/1 -> Convert61/1
  Convert54/1 -> Negate8/1
  Reinterpret44/1 -> Convert54/1
  Negate8/1 -> [x2re_ch3]
  Convert61/1 -> [x2im_ch3]
  [cx_ch3] -> comp_addsub12/2(b), comp_addsub13/1(a)
  Reinterpret40/1 -> Convert59/1
  Convert59/1 -> [x1re_ch3]
  Convert35/1 -> Reinterpret53/1
  Convert36/1 -> Reinterpret75/1
  Convert37/1 -> Reinterpret77/1
  Reinterpret20/1 -> Convert39/1
  Convert38/1 -> Reinterpret20/1
  Convert39/1 -> Reinterpret21/1
  Reinterpret21/1 -> [lamb2x]
  Reinterpret18/1 -> Mux/2
  Reinterpret19/1 -> Mux/3
  [snr] -> Mult/1
  [lamb2x] -> Mult/2
  [lamb1x] -> Delay2/1
  Mult/1 -> Relational3/2
  AddSub/1 -> Register/1
  Register/1 -> AddSub/2, Shift/1
  [doax] -> AddSub/1
  Relational3/1 -> Convert40/1
  AddSub1/1 -> Register1/1
  Register1/1 -> Relational4/2, AddSub1/2
  Constant17/1 -> Relational4/1
  Relational4/1 -> doa_valid/1, Delay22/1, Register1/2, Register/2
  Shift/1 -> doa_acc/1
  [accdone] -> Delay16/1
  Delay16/1 -> Delay20/1
  Delay20/1 -> Delay21/1
  Delay21/1 -> [accdone2]
  [accdone3] -> AddSub/3
  Logical/1 -> [accdone3], AddSub1/3
  [accdone2] -> Delay11/1
  Delay22/1 -> Mux2/1, Logical/2
  Delay2/1 -> Relational3/1
  [co_ch0] -> comp_addsub/1(a), comp_addsub1/2(b)
  Constant6/1 -> sqrt/2
  Reinterpret42/1 -> Delay/1
  tracex3/1 -> Convert26/1
  tracex4/1 -> Convert38/1
  tracex2/1 -> sqrt/1
  Convert1/1 -> Reinterpret42/1
  Reinterpret30/1 -> Convert1/1
  tracex1/1 -> Reinterpret30/1
  Delay/1 -> Delay1/1
  Delay1/1 -> tracex4/1, tracex3/2
  Mult1/1 -> Convert21/1
  Mult3/1 -> Convert11/1
  Mult2/1 -> Convert22/1
  Mult5/1 -> Convert23/1
  Mult4/1 -> Convert72/1
  Reinterpret75/1 -> Convert3/1
  Convert3/1 -> bus_create1/3(in3), Reinterpret76/1
  Reinterpret77/1 -> Convert4/1
  Convert4/1 -> bus_create1/4(in4), Reinterpret10/1
  Convert8/1 -> tracex3/3, tracex4/3
  Reinterpret/1 -> tracex4/2, tracex3/1
  Reinterpret5/1 -> Convert/1
  Reinterpret6/1 -> Mult13/2, Mult13/1
  [x22_ch23] -> Mult11/1
  [gain1] -> Mult7/2, Mult8/2, Mult9/2, Mult10/2, Mult11/2, Mult6/2
  [x2re_ch0] -> Mult3/2
  [x2re_ch0] -> Mult5/2, Mult5/1
  [x1im_ch0] -> Mult2/1
  [x2im_ch0] -> Mult2/2
  [x2im_ch0] -> Mult4/2, Mult4/1
  Subsystem16/1(Outacc) -> Convert9/1, Mult14/1
  Subsystem16/2(Outen) -> corr_mat_red/3(we), Counter/1, Mx22/2, Mx12/2, Mx11/2, freeze_cntr/1, [accdone]
  nacc/1 -> [Nacc]
  en/1 -> edge_detect1/1, [en]
  edge_detect1/1 -> [rst]
  Convert7/1 -> [x2im_ch0]
  Reinterpret53/1 -> Convert2/1
  Convert2/1 -> bus_create1/2(in2), Reinterpret64/1
  Subsystem17/1(Outacc) -> Convert10/1, Mult15/1
  Subsystem5/1(Outacc) -> Convert16/1, Mult16/1
  Reinterpret64/1 -> Mx11/1
  Reinterpret76/1 -> Mx12/1
  Reinterpret10/1 -> Mx22/1
  Mx11/1 -> tracex1/1, Delay4/1, a_c1/1
  Mx22/1 -> a_c1/2, tracex1/2
  Convert/1 -> Reinterpret6/1
  Mx12/1 -> Delay14/1, Mult12/2, Mult12/1
  Mult13/1 -> tracex2/1
  Mult12/1 -> tracex2/2
  a_c1/1 -> Reinterpret5/1
  sqrt/1 -> Reinterpret/1
  sqrt/2 -> Convert8/1
  Delay14/1 -> [bx]
  Constant16/1 -> Relational2/2
  Relational2/1 -> Mux1/1, Mux/1
  Mux/1 -> Black Box/1
  Negate2/1 -> Reinterpret19/1
  Reinterpret14/1 -> Negate3/1, Mux1/2
  Negate3/1 -> Mux1/3
  Mux1/1 -> [doax]
  Mult6/1 -> Convert29/1
  Mult7/1 -> Convert30/1
  Mult8/1 -> Convert33/1
  Mult9/1 -> Convert31/1
  Mult10/1 -> Convert34/1
  Mult11/1 -> Convert32/1
  [x11_ch01] -> Mult6/1
  [x11_ch23] -> Mult7/1
  [x12_ch01] -> Mult8/1
  [x12_ch23] -> Mult9/1
  [x22_ch01] -> Mult10/1
  AddSub14/1 -> AddSub17/1
  AddSub15/1 -> AddSub17/2
  Convert23/1 -> AddSub18/1
  Convert29/1 -> bus_create/3(in3), Subsystem16/1(re)
  Convert30/1 -> bus_create/4(in4), Subsystem16/2(im)
  Convert31/1 -> bus_create/6(in6), Subsystem17/2(im)
  Convert32/1 -> bus_create/8(in8), Subsystem5/2(im)
  Convert33/1 -> bus_create/5(in5), Subsystem17/1(re)
  Convert34/1 -> bus_create/7(in7), Subsystem5/1(re)
  Mult14/1 -> Convert35/1
  Mult15/1 -> Convert36/1
  Mult16/1 -> Convert37/1
  [gain2] -> Mult16/2, Mult15/2, Mult14/2
  AddSub10/1 -> AddSub13/1
  AddSub11/1 -> AddSub13/2
  Convert11/1 -> AddSub8/1
  Convert22/1 -> AddSub8/2
  Convert12/1 -> AddSub9/1
  Convert24/1 -> AddSub9/2
  Convert13/1 -> AddSub10/1
  Convert66/1 -> AddSub10/2
  Convert14/1 -> AddSub11/1
  Convert67/1 -> AddSub11/2
  AddSub18/1 -> AddSub16/1
  AddSub19/1 -> AddSub16/2
  Convert72/1 -> AddSub18/2
  Convert25/1 -> AddSub19/1
  Convert73/1 -> AddSub19/2
  Convert68/1 -> AddSub14/1
  Convert74/1 -> AddSub14/2
  Convert69/1 -> AddSub15/1
  Convert75/1 -> AddSub15/2
  AddSub6/1 -> [x11_ch01]
  AddSub7/1 -> [x11_ch23]
  AddSub12/1 -> [x12_ch01]
  AddSub13/1 -> [x12_ch23]
  AddSub16/1 -> [x22_ch01]
  AddSub17/1 -> [x22_ch23]
  arctan/2 -> Terminator2/1
  comp_addsub4/1(out) -> c_to_ri4/1
  c_to_ri4/1 -> Reinterpret22/1
  c_to_ri4/2 -> Reinterpret23/1
  comp_addsub5/1(out) -> c_to_ri5/1
  c_to_ri5/1 -> Reinterpret7/1
  c_to_ri5/2 -> Reinterpret8/1
  Convert48/1 -> [x2im_ch1]
  [cx_ch1] -> comp_addsub5/1(a), comp_addsub4/2(b)
  Reinterpret22/1 -> Convert46/1
  Convert46/1 -> [x1re_ch1]
  Convert47/1 -> [x1im_ch1]
  Reinterpret23/1 -> Convert47/1
  Reinterpret7/1 -> Convert48/1
  Convert41/1 -> Negate4/1
  Reinterpret8/1 -> Convert41/1
  Negate4/1 -> [x2re_ch1]
  [co_ch3] -> comp_addsub12/1(a), comp_addsub13/2(b)
  comp_addsub12/1(out) -> c_to_ri12/1
  c_to_ri12/1 -> Reinterpret40/1
  c_to_ri12/2 -> Reinterpret41/1
  comp_addsub13/1(out) -> c_to_ri13/1
  [co_ch2] -> comp_addsub9/2(b), comp_addsub8/1(a)
  comp_addsub8/1(out) -> c_to_ri8/1
  c_to_ri8/1 -> Reinterpret37/1
  c_to_ri8/2 -> Reinterpret38/1
  comp_addsub9/1(out) -> c_to_ri9/1
  c_to_ri9/1 -> Reinterpret39/1
  c_to_ri9/2 -> Reinterpret45/1
  Convert64/1 -> [x2im_ch2]
  [cx_ch2] -> comp_addsub9/1(a), comp_addsub8/2(b)
  Reinterpret37/1 -> Convert62/1
  Convert62/1 -> [x1re_ch2]
  Convert63/1 -> [x1im_ch2]
  Reinterpret38/1 -> Convert63/1
  Reinterpret39/1 -> Convert64/1
  Convert49/1 -> Negate6/1
  Reinterpret45/1 -> Convert49/1
  Negate6/1 -> [x2re_ch2]
  [co_ch1] -> comp_addsub5/2(b), comp_addsub4/1(a)
  Delay11/1 -> Logical/1
  Mux2/1 -> AddSub1/1
  Convert40/1 -> Mux2/2
  Constant18/1 -> Mux2/3
  Mult17/1 -> Convert70/1
  [x1re_ch1] -> Mult17/1, Mult17/2
  [x1im_ch1] -> Mult18/1, Mult18/2
  [x1re_ch1] -> Mult20/1
  [x2re_ch1] -> Mult20/2
  [x2re_ch1] -> Mult22/1, Mult22/2
  [x1im_ch1] -> Mult19/1
  [x2im_ch1] -> Mult19/2
  [x2im_ch1] -> Mult21/1, Mult21/2
  Mult18/1 -> Convert83/1
  Mult20/1 -> Convert12/1
  Mult19/1 -> Convert24/1
  Mult22/1 -> Convert25/1
  Mult21/1 -> Convert73/1
  Mult23/1 -> Convert71/1
  [x1re_ch2] -> Mult23/1, Mult23/2
  [x1im_ch2] -> Mult24/1, Mult24/2
  [x1re_ch2] -> Mult32/1
  [x2re_ch2] -> Mult32/2
  [x2re_ch2] -> Mult34/1, Mult34/2
  Black Box/2 -> Terminator/1
  [x1im_ch2] -> Mult28/1
  [x2im_ch2] -> Mult28/2
  [x2im_ch2] -> Mult33/1, Mult33/2
  Mult24/1 -> Convert93/1
  Mult32/1 -> Convert13/1
  Mult28/1 -> Convert66/1
  Mult34/1 -> Convert68/1
  Mult33/1 -> Convert74/1
  Mult25/1 -> Convert82/1
  [x1re_ch3] -> Mult25/2, Mult25/1
  [x1im_ch3] -> Mult26/2, Mult26/1
  [x1re_ch3] -> Mult29/1
  [x2re_ch3] -> Mult29/2
  [x2re_ch3] -> Mult31/2, Mult31/1
  [x1im_ch3] -> Mult27/1
  [x2im_ch3] -> Mult27/2
  [x2im_ch3] -> Mult30/2, Mult30/1
  Mult26/1 -> Convert94/1
  Mult29/1 -> Convert14/1
  Mult27/1 -> Convert67/1
  Mult31/1 -> Convert69/1
  Mult30/1 -> Convert75/1
  Convert20/1 -> AddSub2/1
  Convert21/1 -> AddSub2/2
  Convert70/1 -> AddSub3/1
  Convert83/1 -> AddSub3/2
  Convert71/1 -> AddSub4/1
  Convert93/1 -> AddSub4/2
  Convert82/1 -> AddSub5/1
  Convert94/1 -> AddSub5/2
  AddSub2/1 -> AddSub6/1
  AddSub3/1 -> AddSub6/2
  AddSub4/1 -> AddSub7/1
  AddSub5/1 -> AddSub7/2
  AddSub8/1 -> AddSub12/1
  AddSub9/1 -> AddSub12/2
  comp_addsub/1(out) -> c_to_ri/1
  c_to_ri/1 -> Reinterpret11/1
  c_to_ri/2 -> Reinterpret12/1
  comp_addsub1/1(out) -> c_to_ri1/1
  c_to_ri1/1 -> Reinterpret13/1
  c_to_ri1/2 -> Reinterpret9/1
  [Nacc] -> Subsystem5/3(Nacc), Subsystem17/3(Nacc), Subsystem16/3(Nacc)
  [rst] -> Subsystem5/5(rst), Subsystem17/5(rst), Subsystem16/5(rst)
  Mult0/1 -> Convert20/1
  [en] -> Subsystem5/4(en), Subsystem17/4(en), Subsystem16/4(en)
  [x1re_ch0] -> Mult0/2, Mult0/1
  [x1im_ch0] -> Mult1/2, Mult1/1
  [x1re_ch0] -> Mult3/1
  gain1/1 -> [gain1]
  gain2/1 -> [gain2]
  snr/1 -> [snr]
  a1_ch0/1 -> [co_ch0]
  a1_ch1/1 -> [co_ch1]
  a1_ch2/1 -> [co_ch2]
  a1_ch3/1 -> [co_ch3]
  a2_ch0/1 -> [cx_ch0]
  a2_ch1/1 -> [cx_ch1]
  a2_ch2/1 -> [cx_ch2]
  a2_ch3/1 -> [cx_ch3]
  Constant/1 -> bus_create/2(in2), bus_create/1(in1)
  bus_create/1(bus_out) -> snapshot/1(din)
  Constant1/1 -> snapshot/3(trig), snapshot/2(we)
  Constant2/1 -> bus_create1/1(in1)
  bus_create1/1(bus_out) -> corr_mat_red/2(data_in)
  Counter/1 -> corr_mat_red/1(addr)
  freeze_cntr/1 -> r11/1(addr), r12/1(addr), r1/1(addr)
  freeze_cntr/2 -> r1/3(we), r12/3(we), r11/3(we)
  Convert16/1 -> Reinterpret1/1
  Reinterpret1/1 -> r1/2(data_in)
  Convert10/1 -> Reinterpret2/1
  Reinterpret2/1 -> r12/2(data_in)
  Convert9/1 -> Reinterpret3/1
  Reinterpret3/1 -> r11/2(data_in)
  trig/1(in_reg) -> freeze_cntr/2

== system doa_diego/Subsystem2 ==
  gain : Inport
  sync : Inport  Port=2
  a1_ch0 : Inport  Port=3
  a1_ch1 : Inport  Port=4
  a1_ch2 : Inport  Port=5
  a1_ch3 : Inport  Port=6
  a2_ch0 : Inport  Port=7
  a2_ch1 : Inport  Port=8
  a2_ch2 : Inport  Port=9
  a2_ch3 : Inport  Port=10
  Delay : xbsIndex_r4/Delay  rst=off  infoeditControl=Selection of Reset will increase slice count due to use of real FFs and instead of SRLs  en=off  latency=3  dbl_ovrd=off  reg_retiming=off  block_type=delay
  Subsystem : Subsystem
  Subsystem1 : Subsystem
  Subsystem2 : Subsystem
  Subsystem3 : Subsystem
  Subsystem4 : Subsystem
  Subsystem5 : Subsystem
  Subsystem6 : Subsystem
  Subsystem7 : Subsystem
  sync_out : Outport
  spec0_0 : Outport  Port=2
  spec0_1 : Outport  Port=3
  spec0_2 : Outport  Port=4
  spec0_3 : Outport  Port=5
  spec1_0 : Outport  Port=6
  spec1_1 : Outport  Port=7
  spec1_2 : Outport  Port=8
  spec1_3 : Outport  Port=9
  wires:
  a1_ch1/1 -> Subsystem1/2(In2)
  a1_ch0/1 -> Subsystem/2(In2)
  Subsystem1/1(Out1) -> spec0_1/1
  Subsystem/1(Out1) -> spec0_0/1
  a1_ch2/1 -> Subsystem2/2(In2)
  Subsystem2/1(Out1) -> spec0_2/1
  a1_ch3/1 -> Subsystem3/2(In2)
  Subsystem3/1(Out1) -> spec0_3/1
  a2_ch0/1 -> Subsystem4/2(In2)
  Subsystem4/1(Out1) -> spec1_0/1
  a2_ch1/1 -> Subsystem5/2(In2)
  Subsystem5/1(Out1) -> spec1_1/1
  a2_ch2/1 -> Subsystem6/2(In2)
  Subsystem6/1(Out1) -> spec1_2/1
  a2_ch3/1 -> Subsystem7/2(In2)
  gain/1 -> Subsystem7/1(In1), Subsystem6/1(In1), Subsystem5/1(In1), Subsystem4/1(In1), Subsystem3/1(In1), Subsystem2/1(In1), Subsystem1/1(In1), Subsystem/1(In1)
  Subsystem7/1(Out1) -> spec1_3/1
  sync/1 -> Delay/1
  Delay/1 -> sync_out/1
